simulink model "TosAeb"  (R2021a) (continued)
== system TosAeb/FcwAebJa_Step_sys/ALG_AEBSystem/ALG_AEBController/AEB_FuncImpManagementApp/FuncImpArbCalc/BrkReadyFuncImpArbCalc1 == (continued)
  Bus Selector2/9 -> BrkFuncImpSuppCondCalc/9(brkFuncActiveCond)  "<brkFuncActiveCond>"
  Bus Selector3/1 -> BrkReadyFuncImpArbCalc/1(prefillModStsArb)  "<prefillModStsArb>"
  Bus Selector3/2 -> BrkReadyFuncImpArbCalc/2(ABAModStsArb)  "<ABAModStsArb>"
  Bus Selector3/3 -> BrkReadyFuncImpArbCalc/3(pedPrefillModStsArb)  "<pedPrefillModStsArb>"
  Bus Selector3/4 -> BrkReadyFuncImpArbCalc/4(cycPrefillModStsArb)  "<cycPrefillModStsArb>"
  Bus Selector3/5 -> BrkReadyFuncImpArbCalc/5(JA_vehPrefillModStsArb)  "<JA_vehPrefillModStsArb>"
  Bus Selector3/6 -> BrkReadyFuncImpArbCalc/6(JA_PTW_PrefillModStsArb)  "<JA_PTW_PrefillModStsArb>"
  Bus Selector3/7 -> BrkReadyFuncImpArbCalc/7(JA_cycPrefillModStsArb)  "<JA_cycPrefillModStsArb>"
  Bus Selector3/8 -> BrkReadyFuncImpArbCalc/8(JA_pedPrefillModStsArb)  "<JA_pedPrefillModStsArb>"
  pedABAModStsArb/1 -> Bus Creator4/4  "pedABAModStsArb"
  Bus Selector/4 -> BrkReadyFuncImpAborCondCalc/4(pedABAModStsArb)  "<pedABAModStsArb>"
  BrkReadyFuncImpAborCondCalc/7(JA_vehPrefillFuncImpAborArb) -> Bus Creator/7  "JA_vehPrefillFuncImpAborArb"
  BrkReadyFuncImpAborCondCalc/8(JA_pedPrefillFuncImpAborArb) -> Bus Creator/8  "JA_pedPrefillFuncImpAborArb"
  BrkReadyFuncImpAborCondCalc/9(JA_cycPrefillFuncImpAborArb) -> Bus Creator/9  "JA_cycPrefillFuncImpAborArb"
  BrkReadyFuncImpAborCondCalc/10(JA_PTW_PrefillFuncImpAborArb) -> Bus Creator/10  "JA_PTW_PrefillFuncImpAborArb"
  BrkReadyFuncImpAborCondCalc/11(FCTB_PrefillFuncImpAborArb) -> Bus Creator/11  "FCTB_PrefillFuncImpAborArb"
  BrkReadyFuncImpAborCondCalc/12(FCTB_ABAPrefillFuncImpAborArb) -> Bus Creator/12  "FCTB_ABAPrefillFuncImpAborArb"
  Bus Selector4/1 -> BrkReadyFuncImpArbCalc/9(JA_vehPrefillFuncImpAborCond)  "<JA_vehPrefillFuncImpAborCond>"
  Bus Selector4/2 -> BrkReadyFuncImpArbCalc/10(cycPrefillFuncImpAborCond)  "<cycPrefillFuncImpAborCond>"
  Bus Selector4/3 -> BrkReadyFuncImpArbCalc/11(pedPrefillFuncImpAborCond)  "<pedPrefillFuncImpAborCond>"
  Bus Selector4/4 -> BrkReadyFuncImpArbCalc/12(vehPrefillFuncImpAborCond)  "<vehPrefillFuncImpAborCond>"
  Bus Selector4/5 -> BrkReadyFuncImpArbCalc/13(vehABAFuncImpAborCond)  "<vehABAFuncImpAborCond>"
  Bus Selector4/6 -> BrkReadyFuncImpArbCalc/14(pedABAFuncImpAborCond)  "<pedABAFuncImpAborCond>"
  Bus Selector4/7 -> BrkReadyFuncImpArbCalc/15(JA_vehPrefillFuncImpAborArb)  "<JA_vehPrefillFuncImpAborArb>"
  Bus Selector4/8 -> BrkReadyFuncImpArbCalc/16(JA_pedPrefillFuncImpAborArb)  "<JA_pedPrefillFuncImpAborArb>"
  Bus Selector4/9 -> BrkReadyFuncImpArbCalc/17(JA_cycPrefillFuncImpAborArb)  "<JA_cycPrefillFuncImpAborArb>"
  Bus Selector4/10 -> BrkReadyFuncImpArbCalc/18(JA_PTW_PrefillFuncImpAborArb)  "<JA_PTW_PrefillFuncImpAborArb>"
  Bus Selector4/11 -> BrkReadyFuncImpArbCalc/19(FCTB_PrefillFuncImpAborArb)  "<FCTB_PrefillFuncImpAborArb>"
  Bus Selector4/12 -> BrkReadyFuncImpArbCalc/20(FCTB_ABAPrefillFuncImpAborArb)  "<FCTB_ABAPrefillFuncImpAborArb>"
  BrkFuncImpSuppCondCalc/7(JA_vehPrefillFuncImpSuppCond) -> Bus Creator2/7  "JA_vehPrefillFuncImpSuppCond"
  BrkFuncImpSuppCondCalc/8(JA_pedPrefillFuncImpSuppCond) -> Bus Creator2/8  "JA_pedPrefillFuncImpSuppCond"
  BrkFuncImpSuppCondCalc/9(JA_cycPrefillFuncImpSuppCond) -> Bus Creator2/9  "JA_cycPrefillFuncImpSuppCond"
  BrkFuncImpSuppCondCalc/10(JA_PTW_PrefillFuncImpSuppCond) -> Bus Creator2/10  "JA_PTW_PrefillFuncImpSuppCond"
  BrkFuncImpSuppCondCalc/11(FCTB_PrefillFuncImpSuppCond) -> Bus Creator2/11  "FCTB_PrefillFuncImpSuppCond"
  BrkFuncImpSuppCondCalc/12(FCTB_ABAPrefillFuncImpSuppCond) -> Bus Creator2/12  "FCTB_ABAPrefillFuncImpSuppCond"

== system TosAeb/FcwAebJa_Step_sys/ALG_AEBSystem/ALG_AEBController/AEB_FuncImpManagementApp/FuncImpArbCalc/WarningFuncImpArbCalc ==
  AEBModStsArb : Inport
  brkReadyFuncActvCond : Inport  Port=2
  brkFuncActvCond : Inport  Port=3
  Bus Creator : BusCreator  Inputs=4
  Bus Creator1 : BusCreator  Inputs=4
  Bus Creator2 : BusCreator  Inputs=4
  Bus Creator3 : BusCreator  Inputs=2
  Bus Selector : BusSelector  OutputSignals=latentWarningFuncImpArb,preWarningFuncImpArb,acuteWarningFuncImpArb,pedPreWarningFuncImpArb
  WarningFuncImpAborCond : Subsystem
  WarningFuncImpArbCalc : Subsystem
  WarningFuncImpSuppCond : Subsystem
  warningFuncActvCondCalc : Subsystem
  warningFuncActvCond : Outport
  warningFuncImpSuppCondAbor : Outport  Port=2
  warningFuncImpArb : Outport  Port=3
  wires:
  Bus Creator3/1 -> warningFuncImpSuppCondAbor/1
  Bus Creator2/1 -> Bus Creator3/2  "warningFuncImpSuppCond"
  WarningFuncImpSuppCond/1(latentWarningFuncImpSuppCond) -> Bus Creator2/1  "vehLatentWarningFuncImpSuppCond"
  WarningFuncImpSuppCond/2(pedWarningFuncImpSuppCond) -> Bus Creator2/2  "pedPreWarningFuncImpSuppCond"
  WarningFuncImpSuppCond/3(preWarningFuncImpSuppCond) -> Bus Creator2/3  "vehPreWarningFuncImpSuppCond"
  WarningFuncImpSuppCond/4(acuteWarningFuncImpSuppCond) -> Bus Creator2/4  "vehAcuteWarningFuncimpSuppCond"
  WarningFuncImpAborCond/4(acuteWarningFuncImpAborCond) -> Bus Creator/4  "vehAcuteWarningFuncimpAborCond"
  WarningFuncImpAborCond/3(preWarningFuncImpAborCond) -> Bus Creator/3  "vehPreWarningFuncImpAborCond"
  WarningFuncImpAborCond/2(pedWarningFuncImpAborCond) -> Bus Creator/2  "pedPreWarningFuncImpAborCond"
  WarningFuncImpAborCond/1(latentWarningFuncImpAborCond) -> Bus Creator/1  "vehLatentWarningFuncImpAborCond"
  warningFuncActvCondCalc/1(warningFuncActvCond) -> warningFuncActvCond/1
  Bus Selector/4 -> warningFuncActvCondCalc/4(pedPreWarningFuncImpArb)  "<pedPreWarningFuncImpArb>"
  Bus Selector/3 -> warningFuncActvCondCalc/3(acuteWarningFuncImpArb)  "<acuteWarningFuncImpArb>"
  Bus Selector/2 -> warningFuncActvCondCalc/2(preWarningFuncImpArb)  "<preWarningFuncImpArb>"
  Bus Selector/1 -> warningFuncActvCondCalc/1(latentWarningFuncImpArb)  "<latentWarningFuncImpArb>"
  Bus Creator1/1 -> warningFuncImpArb/1, Bus Selector/1  "warningFuncImpArb"
  WarningFuncImpArbCalc/1(latentWarningFuncImpArb) -> Bus Creator1/1  "latentWarningFuncImpArb"
  WarningFuncImpArbCalc/2(preWarningFuncImpArb) -> Bus Creator1/2  "preWarningFuncImpArb"
  WarningFuncImpArbCalc/4(pedPreWarningFuncImpArb) -> Bus Creator1/4  "pedPreWarningFuncImpArb"
  WarningFuncImpArbCalc/3(acuteWarningFuncImpArb) -> Bus Creator1/3  "acuteWarningFuncImpArb"
  Bus Creator/1 -> WarningFuncImpArbCalc/2(warningFuncImpAborCondCond), Bus Creator3/1  "warningFuncImpAborCond"
  brkFuncActvCond/1 -> WarningFuncImpSuppCond/1(brkFuncActvCond), WarningFuncImpAborCond/3(brkFuncActvCond)
  brkReadyFuncActvCond/1 -> WarningFuncImpSuppCond/2(brkReadyFuncActvCond), WarningFuncImpAborCond/2(brkReadyFuncActvCond)
  AEBModStsArb/1 -> WarningFuncImpSuppCond/3(AEBModStsArb), WarningFuncImpAborCond/1(AEBModStsArb), WarningFuncImpArbCalc/1(AEBModStsArb)

== system TosAeb/FcwAebJa_Step_sys/ALG_AEBSystem/ALG_AEBController/AEB_FuncImpManagementApp/FuncManeuArbCalc/BrkReadyManeuArbCalc ==
  breakReadyFuncImpArb : Inport
  ABPAvailable : Inport  Port=2
  ABAAvailable : Inport  Port=3
  longCollisionSeverity : Inport  Port=4
  pedCollisionSeverity : Inport  Port=5
  ABPActive : Inport  Port=6
  ABAActive : Inport  Port=7
  FCTB_ABA_Level : Inport  Port=8
  BrkReadyManeuAbor : Subsystem
  BrkReadyTriggerJudge : Subsystem
  ABPTrigger : Outport
  ABATrigger : Outport  Port=2
  ABALvl : Outport  Port=3
  ABPFuncManeuAbor : Outport  Port=4
  ABAFuncManeuAbor : Outport  Port=5
  wires:
  FCTB_ABA_Level/1 -> BrkReadyTriggerJudge/6(FCTB_ABA_Level)
  longCollisionSeverity/1 -> BrkReadyTriggerJudge/4(longCollisionSeverity)
  BrkReadyTriggerJudge/3(ABALvl) -> ABALvl/1
  BrkReadyManeuAbor/2(ABAFuncManeuAbor) -> ABAFuncManeuAbor/1
  BrkReadyManeuAbor/1(ABPFuncManeuAbor) -> ABPFuncManeuAbor/1
  ABAActive/1 -> BrkReadyManeuAbor/4(ABAActive)
  ABPActive/1 -> BrkReadyManeuAbor/3(ABPActive)
  BrkReadyTriggerJudge/2(ABATrigger) -> ABATrigger/1, BrkReadyManeuAbor/2(ABATrigger)
  BrkReadyTriggerJudge/1(ABPTrigger) -> ABPTrigger/1, BrkReadyManeuAbor/1(ABPTrigger)
  pedCollisionSeverity/1 -> BrkReadyTriggerJudge/5(pedCollisionSeverity)
  ABAAvailable/1 -> BrkReadyTriggerJudge/3(ABAAvailable)
  ABPAvailable/1 -> BrkReadyTriggerJudge/2(ABPAvailable)
  breakReadyFuncImpArb/1 -> BrkReadyTriggerJudge/1(breakReadyFuncImpArb)

== system TosAeb/FcwAebJa_Step_sys/ALG_AEBSystem/ALG_AEBController/AEB_FuncImpManagementApp/FuncManeuArbCalc/WarningManeuArbCalc ==
  AEBModStsFuncImpArb : Inport
  subjVel : Inport  Port=2
  AWBActive : Inport  Port=3
  WarningAborCalc : Subsystem
  WarningTriggerCalc : Subsystem
  latentWarningTrigger : Outport
  preWarningTrigger : Outport  Port=2
  acuteWarningTrigger : Outport  Port=3
  acuteWarningLvl : Outport  Port=4
  acuteWarningFuncManeuAbor : Outport  Port=5
  wires:
  WarningAborCalc/1(acuteWarningFuncManeuAbor) -> acuteWarningFuncManeuAbor/1
  AWBActive/1 -> WarningAborCalc/2(AWBActive)
  WarningTriggerCalc/4(acuteWarningLvl) -> acuteWarningLvl/1
  WarningTriggerCalc/3(acuteWarningTrigger) -> acuteWarningTrigger/1
  WarningTriggerCalc/2(preWarningTrigger) -> preWarningTrigger/1
  WarningTriggerCalc/1(latentWarningTrigger) -> WarningAborCalc/1(acuteWarningTrigger), latentWarningTrigger/1
  subjVel/1 -> WarningTriggerCalc/2(subjVel)
  AEBModStsFuncImpArb/1 -> WarningTriggerCalc/1(AEBModStsFuncImpArb)

== system TosAeb/FcwAebJa_Step_sys/ALG_AEBSystem/ALG_AEBController/AEB_FuncImpManagementApp/FuncManeuArbCalc/brkFuncManeuArbCalc ==
  brkFuncImpArb : Inport
  AEBAvailable : Inport  Port=2
  ObjectMovingState : Inport  Port=3
  accelAvoid : Inport  Port=4
  axAbsObj : Inport  Port=5
  AEBBA : Inport  Port=6
  AEBIB : Inport  Port=7
  subjVel : Inport  Port=8  OutDataTypeStr=single
  vx : Inport  Port=9
  longObjCalcInfo : Inport  Port=10  OutDataTypeStr=Bus: BusLongObjCalcInfo
  VehicleStandstill : Inport  Port=11
  VSC_VehStandstill : Inport  Port=12  OutDataTypeStr=boolean
  FCTB_BrkgReqVal : Inport  Port=13
  subjLongAccel : Inport  Port=14
  AEBTriggerStateCalc : Subsystem
  Bus Selector : BusSelector  OutputSignals=EBAFuncImpArb
  Bus Selector1 : BusSelector  OutputSignals=LongObjRawInfo.ObjectMovingState,LongObjRawInfo.vx,LongObjRawInfo.ax,longObjAbsInfo.dxFrontBumper,longObjAbsInfo.axAbsObj,longObjAbsInfo.vxAbsObj
  axTgtReqValueCalc1 : Subsystem
  axTgtReqValueManeuCalc : Subsystem
  brkManeuAborCalc : Subsystem
  AEBTrigger : Outport
  AEBTriggerManeu : Outport  Port=2
  axTgtReqValueManeu : Outport  Port=3
  vehBrkManeuAbor : Outport  Port=4
  latBrkManeuAbor : Outport  Port=5
  JABrkManeuAbor : Outport  Port=6
  wires:
  Bus Selector1/5 -> axTgtReqValueCalc1/11(axAbsObj)  "<axAbsObj>"
  Bus Selector1/4 -> axTgtReqValueCalc1/10(dxFrontBumper)  "<dxFrontBumper>"
  Bus Selector1/2 -> axTgtReqValueCalc1/8(vx)  "<vx>"
  Bus Selector1/3 -> axTgtReqValueCalc1/9(ax)  "<ax>"
  Bus Selector1/1 -> axTgtReqValueCalc1/7(ObjectMovingState)  "<ObjectMovingState>"
  longObjCalcInfo/1 -> Bus Selector1/1
  subjLongAccel/1 -> axTgtReqValueCalc1/6(subjLongAccel)
  subjVel/1 -> [subjVel], axTgtReqValueCalc1/5(subjVel)
  VehicleStandstill/1 -> axTgtReqValueCalc1/3(VehicleStandstill)
  FCTB_BrkgReqVal/1 -> axTgtReqValueCalc1/2(FCTB_BrkgReqVal)
  axTgtReqValueCalc1/1(FctbIsNeedBrake) -> AEBTriggerStateCalc/3(FctbIsNeedBrake)
  axTgtReqValueCalc1/2(axTgtReqValue) -> axTgtReqValueManeuCalc/2(axTgtReqValue)  "axTgtReqValue"
  vx/1 -> brkManeuAborCalc/9(vx)
  AEBAvailable/1 -> AEBTriggerStateCalc/1(AEBAvailable)
  Bus Selector/1 -> brkManeuAborCalc/3(EBAFuncSts)  "<EBAFuncImpArb>"
  [AEBModStsFuncImpArb] -> Bus Selector/1
  [subjVel] -> brkManeuAborCalc/2(subjVel)
  brkManeuAborCalc/3(JABrkManeuAbor) -> JABrkManeuAbor/1
  brkManeuAborCalc/2(latBrkManeuAbor) -> latBrkManeuAbor/1
  brkManeuAborCalc/1(vehBrkManeuAbor) -> vehBrkManeuAbor/1
  ObjectMovingState/1 -> brkManeuAborCalc/5(ObjectMovingState)
  axTgtReqValueManeuCalc/2(axTgtReqValueManeu) -> axTgtReqValueManeu/1
  AEBIB/1 -> brkManeuAborCalc/8(AEBIB)
  AEBBA/1 -> brkManeuAborCalc/7(AEBBA)
  accelAvoid/1 -> brkManeuAborCalc/4(accelAvoid)
  axAbsObj/1 -> brkManeuAborCalc/6(axAbsObj)
  AEBTriggerStateCalc/1(AEBTrigger) -> AEBTrigger/1, axTgtReqValueManeuCalc/1(AEBTrigger), brkManeuAborCalc/1(AEBTrigger)
  brkFuncImpArb/1 -> axTgtReqValueCalc1/1(AEBModStsFuncImpArb), [AEBModStsFuncImpArb], AEBTriggerStateCalc/2(brkFuncImpArb)
  axTgtReqValueManeuCalc/1(AEBTriggerManeu) -> AEBTriggerManeu/1
  VSC_VehStandstill/1 -> axTgtReqValueCalc1/4(VSC_VehStandstill)  "VSC_VehStandstill"
  Bus Selector1/6 -> axTgtReqValueCalc1/12(vxAbsObj)  "<vxAbsObj>"

== system TosAeb/FcwAebJa_Step_sys/ALG_AEBSystem/ALG_AEBController/AEB_JaObjCalcApp/JAPedTgtInfoCalc/JAPedCollisonAssessInfo ==
  ObjectID : Inport
  vx : Inport  Port=2
  vy : Inport  Port=3
  dx : Inport  Port=4
  dy : Inport  Port=5
  dxFrontBumper : Inport  Port=6
  tPredictTime : Inport  Port=7
  subjYawRate : Inport  Port=8
  subjVel : Inport  Port=9
  subjLongAccel : Inport  Port=10
  If : If  IfExpression=u1
  LatPedCollisonAssessInfoCalc : Subsystem  TreatAsAtomicUnit=on
  LatPedCollisonAssessInfoDefault : Subsystem  TreatAsAtomicUnit=on
  Merge : Merge
  ObjIsInvalidJudge : Subsystem
  JAPedCollisonAssessInfo : Outport  OutDataTypeStr=Bus: BusAEBJAPedCollisonAssessInfo
  wires:
  ObjectID/1 -> ObjIsInvalidJudge/1(ObjectID)
  Merge/1 -> JAPedCollisonAssessInfo/1
  LatPedCollisonAssessInfoDefault/1(JAPedCollisonAssessInfo) -> Merge/1
  If/2 -> LatPedCollisonAssessInfoCalc/Action
  LatPedCollisonAssessInfoCalc/1(JAPedCollisonAssessInfo) -> Merge/2
  If/1 -> LatPedCollisonAssessInfoDefault/Action
  ObjIsInvalidJudge/1(ObjIsInvalid) -> If/1
  vx/1 -> LatPedCollisonAssessInfoCalc/1(vx)
  vy/1 -> LatPedCollisonAssessInfoCalc/2(vy)
  dx/1 -> LatPedCollisonAssessInfoCalc/3(dx)
  dy/1 -> LatPedCollisonAssessInfoCalc/4(dy)
  dxFrontBumper/1 -> LatPedCollisonAssessInfoCalc/5(dxFrontBumper)
  tPredictTime/1 -> LatPedCollisonAssessInfoCalc/6(tPredictTime)
  subjYawRate/1 -> LatPedCollisonAssessInfoCalc/7(subjYawRate)
  subjVel/1 -> LatPedCollisonAssessInfoCalc/8(subjVel1)
  subjLongAccel/1 -> LatPedCollisonAssessInfoCalc/9(subjLongAccel)

== system TosAeb/FcwAebJa_Step_sys/ALG_AEBSystem/ALG_AEBController/AEB_JaObjCalcApp/JAPedTgtInfoCalc/JAPedObjCalcInfoCalc ==
  BusAEBJaObj : Inport  OutDataTypeStr=Bus: BusAEBJAObjBus  PortDimensions=1  Partition=on  PartitionDimension=1  PartitionWidth=1  PartitionOffset=0
  BusSubjInfo : Inport  Port=2  OutDataTypeStr=Bus: BusSubjInfo  PortDimensions=1
  Compare To Constant : simulink/Logic and Bit Operations/Compare To Constant  relop===  const=FALSE  OutDataTypeStr=boolean
  For Each : ForEach  DisableCoverage=on
  If : If  IfExpression=u1
  JAPedObjInfoCalc : Subsystem
  JAPedObjInfoDefault : Subsystem  TreatAsAtomicUnit=on
  Merge : Merge
  ObjPreSelectCondCalc : Subsystem  TreatAsAtomicUnit=on
  JAPedObjCalcInfo : Outport  OutDataTypeStr=Bus: BusAEBJAPedObjCalcInfo  ConcatenationDimension=1
  ObjPreSelectCond : Outport  Port=2  ConcatenationDimension=1
  wires:
  If/2 -> JAPedObjInfoCalc/Action
  JAPedObjInfoDefault/1(JAPedObjCalcInfo) -> Merge/1
  ObjPreSelectCondCalc/1(ObjPreSelectCond) -> ObjPreSelectCond/1, Compare To Constant/1
  BusSubjInfo/1 -> JAPedObjInfoCalc/2(subjInfo)
  Merge/1 -> JAPedObjCalcInfo/1
  JAPedObjInfoCalc/1("JAPedObjCalcInfo ") -> Merge/2
  BusAEBJaObj/1 -> JAPedObjInfoCalc/1(AEBJAVehObj), ObjPreSelectCondCalc/1(BusAEBJaObj)
  If/1 -> JAPedObjInfoDefault/Action
  Compare To Constant/1 -> If/1

== system TosAeb/FcwAebJa_Step_sys/ALG_AEBSystem/ALG_AEBController/AEB_JaObjCalcApp/JAPedTgtInfoCalc/TargetSelectCalc ==
  JAPedObjCalcInfo : Inport  OutDataTypeStr=Bus: BusAEBJAPedObjCalcInfo
  ObjPreSelectCond : Inport  Port=2
  subjVel : Inport  Port=3
  SteerWheelAngle_Deg : Inport  Port=4
  JAPedPrefillModSts : Inport  Port=5
  JAPedAEBfullModSts : Inport  Port=6
  tgtSelectFlow : Subsystem
  JAPedTgtObjInfo : Outport
  wires:
  ObjPreSelectCond/1 -> tgtSelectFlow/2(ObjPreSelectCondFufilled)
  JAPedObjCalcInfo/1 -> tgtSelectFlow/1(JAPedObjCalcInfo)
  JAPedPrefillModSts/1 -> tgtSelectFlow/5(JAPedPrefillModSts)
  subjVel/1 -> tgtSelectFlow/3(subjVel)
  JAPedAEBfullModSts/1 -> tgtSelectFlow/6(JAPedAEBfullModSts)
  tgtSelectFlow/1(JAPedTgtObjInfo) -> JAPedTgtObjInfo/1
  SteerWheelAngle_Deg/1 -> tgtSelectFlow/4(SteerWheelAngle_Deg)

== system TosAeb/FcwAebJa_Step_sys/ALG_AEBSystem/ALG_AEBController/AEB_JaObjCalcApp/JAVehTgtInfoCalc/AEBJAVehObjCalc ==
  AEBJaVehObj : Inport  Partition=on  PartitionDimension=1  PartitionWidth=1  PartitionOffset=0
  subjInfo : Inport  Port=2
  Bus Selector : BusSelector  OutputSignals=subjVel
  Compare To Constant : simulink/Logic and Bit Operations/Compare To Constant  relop===  const=FALSE  OutDataTypeStr=boolean
  For Each : ForEach  DisableCoverage=on
  If : If  IfExpression=u1
  JAVehObjCalcInfoDefault : Subsystem  TreatAsAtomicUnit=on
  JAVehObjInfoCalc : Subsystem
  Merge : Merge
  ObjPreSelectCondCalc : Subsystem  TreatAsAtomicUnit=on
  JaVehObjCalcInfo : Outport  OutDataTypeStr=Bus: BusAEBJAVehObjCalcInfo  ConcatenationDimension=1
  ObjPreSelectCond : Outport  Port=2  ConcatenationDimension=1
  wires:
  AEBJaVehObj/1 -> ObjPreSelectCondCalc/1(AEBJaVehObj), JAVehObjInfoCalc/2(AEBJAVehObj)
  subjInfo/1 -> JAVehObjInfoCalc/1(subjInfo), Bus Selector/1
  If/2 -> JAVehObjInfoCalc/Action
  If/1 -> JAVehObjCalcInfoDefault/Action
  JAVehObjInfoCalc/1(JAVehObjCalcInfo) -> Merge/2
  JAVehObjCalcInfoDefault/1(JAVehObjCalcInfo) -> Merge/1
  Merge/1 -> JaVehObjCalcInfo/1
  ObjPreSelectCondCalc/1(ObjPreSelectCondFufilled) -> Compare To Constant/1, ObjPreSelectCond/1
  Bus Selector/1 -> ObjPreSelectCondCalc/2(subjVel)  "<subjVel>"
  Compare To Constant/1 -> If/1

== system TosAeb/FcwAebJa_Step_sys/ALG_AEBSystem/ALG_AEBController/AEB_JaObjCalcApp/JAVehTgtInfoCalc/JAVehCollisonAssessInfoCalc ==
  ObjectID : Inport
  vx : Inport  Port=2
  vy : Inport  Port=3
  dx : Inport  Port=4
  dy : Inport  Port=5
  dxFrontBumper : Inport  Port=6
  tPredictTime : Inport  Port=7
  subjYawRate : Inport  Port=8
  subjVel : Inport  Port=9
  subjLongAccel : Inport  Port=10
  If : If  IfExpression=u1
  JaVehCollisonAssessInfoCalc : Subsystem  TreatAsAtomicUnit=on
  JaVehCollisonAssessInfoDefault : Subsystem  TreatAsAtomicUnit=on
  Merge : Merge
  ObjIsInvalidJudge : Subsystem
  JAVehCollisonAssessInfo : Outport
  wires:
  ObjectID/1 -> ObjIsInvalidJudge/1(ObjectID)
  ObjIsInvalidJudge/1(ObjIsInvalid) -> If/1
  Merge/1 -> JAVehCollisonAssessInfo/1
  If/1 -> JaVehCollisonAssessInfoDefault/Action
  JaVehCollisonAssessInfoDefault/1(JAVehCollisonAssessInfo) -> Merge/1
  dy/1 -> JaVehCollisonAssessInfoCalc/4(dy)
  tPredictTime/1 -> JaVehCollisonAssessInfoCalc/6(tPredictTime)
  subjVel/1 -> JaVehCollisonAssessInfoCalc/8(subjVel)
  subjLongAccel/1 -> JaVehCollisonAssessInfoCalc/9(subjLongAccel)
  dxFrontBumper/1 -> JaVehCollisonAssessInfoCalc/5(dxFrontBumper)
  vy/1 -> JaVehCollisonAssessInfoCalc/2(vy)
  subjYawRate/1 -> JaVehCollisonAssessInfoCalc/7(subjYawRate)
  dx/1 -> JaVehCollisonAssessInfoCalc/3(dx)
  vx/1 -> JaVehCollisonAssessInfoCalc/1(vx)
  If/2 -> JaVehCollisonAssessInfoCalc/Action
  JaVehCollisonAssessInfoCalc/1(JAVehCollisonAssessInfo) -> Merge/2

== system TosAeb/FcwAebJa_Step_sys/ALG_AEBSystem/ALG_AEBController/AEB_JaObjCalcApp/JAVehTgtInfoCalc/TargetFinalSelect ==
  JAVehObjCalcInfo : Inport
  ObjPreSelectCond : Inport  Port=2
  subjVel : Inport  Port=3
  SteerWheelAngle_Deg : Inport  Port=4
  JAVehPrefillModSts : Inport  Port=5
  JAVehAEBfullModSts : Inport  Port=6
  tgtSelect : Subsystem
  JAVehTgtObjInfo : Outport
  wires:
  JAVehObjCalcInfo/1 -> tgtSelect/1(JAVehObjCalcInfo)
  tgtSelect/1(JAVehTgtObjInfo) -> JAVehTgtObjInfo/1
  ObjPreSelectCond/1 -> tgtSelect/2(ObjPreSelectCondFufilled)
  JAVehPrefillModSts/1 -> tgtSelect/5(JAVehPrefillModSts)
  JAVehAEBfullModSts/1 -> tgtSelect/6(JAVehAEBfullModSts)
  SteerWheelAngle_Deg/1 -> tgtSelect/4(SteerWheelAngle_Deg)
  subjVel/1 -> tgtSelect/3(subjVel)

== system TosAeb/FcwAebJa_Step_sys/ALG_AEBSystem/ALG_AEBController/AEB_JaObjCalcApp/JaCycTgtInfoCalc/JaCycCollisonAssessInfo ==
  ObjectID : Inport
  vx : Inport  Port=2
  vy : Inport  Port=3
  dx : Inport  Port=4
  dy : Inport  Port=5
  dxFrontBumper : Inport  Port=6
  tPredictTime : Inport  Port=7
  subjYawRate : Inport  Port=8
  subjVel : Inport  Port=9
  subjLongAccel : Inport  Port=10
  If : If  IfExpression=u1
  JaCycCollisonAssessInfoCalc : Subsystem  TreatAsAtomicUnit=on
  JaCycCollisonAssessInfoDefault : Subsystem  TreatAsAtomicUnit=on
  Merge : Merge
  ObjIsInvalidJudge : Subsystem
  JaCycCollisonAssessInfo : Outport  OutDataTypeStr=Bus: BusAEBJaCycCollisonAssessInfo
  wires:
  ObjectID/1 -> ObjIsInvalidJudge/1(ObjectID)
  Merge/1 -> JaCycCollisonAssessInfo/1
  JaCycCollisonAssessInfoDefault/1(JaCycCollisonAssessInfo) -> Merge/1
  If/2 -> JaCycCollisonAssessInfoCalc/Action
  JaCycCollisonAssessInfoCalc/1(JaCycCollisonAssessInfo) -> Merge/2
  If/1 -> JaCycCollisonAssessInfoDefault/Action
  ObjIsInvalidJudge/1(ObjIsInvalid) -> If/1
  vx/1 -> JaCycCollisonAssessInfoCalc/1(vx)
  vy/1 -> JaCycCollisonAssessInfoCalc/2(vy)
  dx/1 -> JaCycCollisonAssessInfoCalc/3(dx)
  dy/1 -> JaCycCollisonAssessInfoCalc/4(dy)
  dxFrontBumper/1 -> JaCycCollisonAssessInfoCalc/5(dxFrontBumper)
  tPredictTime/1 -> JaCycCollisonAssessInfoCalc/6(tPredictTime)
  subjYawRate/1 -> JaCycCollisonAssessInfoCalc/7(subjYawRate)
  subjVel/1 -> JaCycCollisonAssessInfoCalc/8(subjVel)
  subjLongAccel/1 -> JaCycCollisonAssessInfoCalc/9(subjLongAccel)

== system TosAeb/FcwAebJa_Step_sys/ALG_AEBSystem/ALG_AEBController/AEB_JaObjCalcApp/JaCycTgtInfoCalc/JaCycObjCalcInfoCalc ==
  JaObj : Inport  OutDataTypeStr=Bus: BusAEBJAObjBus  PortDimensions=1  Partition=on  PartitionDimension=1  PartitionWidth=1  PartitionOffset=0
  subjInfo : Inport  Port=2  OutDataTypeStr=Bus: BusSubjInfo  PortDimensions=1
  Compare To Constant : simulink/Logic and Bit Operations/Compare To Constant  relop===  const=FALSE  OutDataTypeStr=boolean
  For Each : ForEach  DisableCoverage=on
  If : If  IfExpression=u1
  JaCycObjInfoCalc : Subsystem
  JaCycObjInfoDefault : Subsystem  TreatAsAtomicUnit=on
  Merge : Merge
  ObjPreSelectCondCalc : Subsystem  TreatAsAtomicUnit=on
  JaCycObjCalcInfo : Outport  OutDataTypeStr=Bus: BusAEBJaCycObjCalcInfo  ConcatenationDimension=1
  ObjPreSelectCond : Outport  Port=2  ConcatenationDimension=1
  wires:
  If/2 -> JaCycObjInfoCalc/Action
  JaCycObjInfoDefault/1(JaCycObjCalcInfo) -> Merge/1
  ObjPreSelectCondCalc/1(ObjPreSelectCond) -> ObjPreSelectCond/1, Compare To Constant/1
  subjInfo/1 -> JaCycObjInfoCalc/2(subjInfo)
  Merge/1 -> JaCycObjCalcInfo/1
  JaObj/1 -> JaCycObjInfoCalc/1(JaCycObj), ObjPreSelectCondCalc/1(JaObj)
  If/1 -> JaCycObjInfoDefault/Action
  Compare To Constant/1 -> If/1
  JaCycObjInfoCalc/1("JaCycObjCalcInfo ") -> Merge/2

== system TosAeb/FcwAebJa_Step_sys/ALG_AEBSystem/ALG_AEBController/AEB_JaObjCalcApp/JaCycTgtInfoCalc/TargetSelectCalc ==
  JaCycObjCalcInfo : Inport  OutDataTypeStr=Bus: BusAEBJaCycObjCalcInfo
  ObjPreSelectCond : Inport  Port=2
  subjVel : Inport  Port=3
  SteerWheelAngle_Deg : Inport  Port=4
  JaCycPrefillModSts : Inport  Port=5
  JaCycAEBfullModSts : Inport  Port=6
  tgtSelectFlow : Subsystem
  JaCycTgtObjInfo : Outport
  wires:
  ObjPreSelectCond/1 -> tgtSelectFlow/2(ObjPreSelectCondFufilled)
  JaCycObjCalcInfo/1 -> tgtSelectFlow/1(JaCycObjCalcInfo)
  JaCycPrefillModSts/1 -> tgtSelectFlow/5(JaCycPrefillModSts)
  subjVel/1 -> tgtSelectFlow/3(subjVel)
  JaCycAEBfullModSts/1 -> tgtSelectFlow/6(JaCycAEBfullModSts)
  tgtSelectFlow/1(JaCycTgtObjInfo) -> JaCycTgtObjInfo/1
  SteerWheelAngle_Deg/1 -> tgtSelectFlow/4(SteerWheelAngle_Deg)

== system TosAeb/FcwAebJa_Step_sys/ALG_AEBSystem/ALG_AEBController/AEB_LatObjInfoCalcApp/LatCycObjCalc/LatCycCollisonAssessInfoCalc ==
  ObjectID : Inport
  cycColliisonRiskProb : Inport  Port=2
  subLongAccel : Inport  Port=3
  subjVel : Inport  Port=4
  If : If  IfExpression=u1
  LatCycCollisonAssessInfoCalc : Subsystem
  LatCycCollisonAssessInfoDefault : Subsystem  TreatAsAtomicUnit=on
  Merge : Merge
  ObjIsInvalidJudge : Subsystem
  latCycCollisonAssessInfo : Outport  OutDataTypeStr=Bus: BusLatCycCollisionAssessInfo
  wires:
  LatCycCollisonAssessInfoCalc/1(latCollisonAssessInfo) -> Merge/2
  Merge/1 -> latCycCollisonAssessInfo/1  "latCycCollisonAssessInfo"
  If/2 -> LatCycCollisonAssessInfoCalc/Action
  If/1 -> LatCycCollisonAssessInfoDefault/Action
  LatCycCollisonAssessInfoDefault/1(latCollisonAssessInfo) -> Merge/1
  ObjIsInvalidJudge/1(ObjIsInvalid) -> If/1
  cycColliisonRiskProb/1 -> LatCycCollisonAssessInfoCalc/2(cycColliisonRiskProb)
  subjVel/1 -> LatCycCollisonAssessInfoCalc/4(subjVel)
  subLongAccel/1 -> LatCycCollisonAssessInfoCalc/3(subLongAccel)
  ObjectID/1 -> LatCycCollisonAssessInfoCalc/1(ObjectID), ObjIsInvalidJudge/1(ObjectID)
